simulink model "Demo_TrafficFlow_cs"  (Simulink 7.6)
settings: Solver=ode5  SolverMode=SingleTasking  StartTime=0.0  StopTime=60  FixedStep=1/20  RelTol=1e-3
PreLoadFcn:
  | check_NUTS_defined
  | try
  |     evalin('base', 'NUTS_defined;');
  | catch
  |     warningMsg = sprintf('PreScan Warning: \nUsing this model requires that MATLAB is configured for PreScan. The model may not display correctly.\n\n ==> Please open MATLAB with the dedicated buttons provided with PreScan');
  |     disp(warningMsg)
  |     warndlg(warningMsg,'WindowStyle','Modal');
  | end
  | 
  | model_closed = 0;
  | assignin('base',['model_closed_' bdroot],false);
InitFcn:
  | PreScanExperimentInitFcn(bdroot)
StopFcn:
  | PreScanExperimentStopFcn(bdroot)
undefined symbols (not defined in the file or in its folder): Advised_Speed_L1, Advised_Speed_L2, Advised_Speed_L3, DiscIntNeverNeededParam, spare_10, spare_5, spare_6, spare_7, spare_8, spare_9, State_L2, Throughput_L1, Throughput_L2, Throughput_L3, Velocity_L1, Velocity_L2, Velocity_L3
libraries: simulink x58, PreScanSimulinkLibrary x40, simulink_extras x32, PreScanUsefulBlocks x22, simulink_need_slupdate x3
(part 2 of 20)

== system Demo_TrafficFlow_cs/Antenna_Rx_2 ==
  Selector : Selector  IndexMode=Zero-based  IndexOptions=Starting index (dialog)  Indices=PortIndex  OutputSizes=PortWidth(1)
  U : Outport
  wires:
  Selector/1 -> U/1
  [SimCoreSensors] -> Selector/1

== system Demo_TrafficFlow_cs/Antenna_Rx_3 ==
  Selector : Selector  IndexMode=Zero-based  IndexOptions=Starting index (dialog)  Indices=PortIndex  OutputSizes=PortWidth(1)
  U : Outport
  wires:
  Selector/1 -> U/1
  [SimCoreSensors] -> Selector/1

== system Demo_TrafficFlow_cs/Antenna_Rx_4 ==
  Selector : Selector  IndexMode=Zero-based  IndexOptions=Starting index (dialog)  Indices=PortIndex  OutputSizes=PortWidth(1)
  U : Outport
  wires:
  Selector/1 -> U/1
  [SimCoreSensors] -> Selector/1

== system Demo_TrafficFlow_cs/Antenna_Rx_7 ==
  Selector : Selector  IndexMode=Zero-based  IndexOptions=Starting index (dialog)  Indices=PortIndex  OutputSizes=PortWidth(1)
  U : Outport
  wires:
  Selector/1 -> U/1
  [SimCoreSensors] -> Selector/1

== system Demo_TrafficFlow_cs/Antenna_Rx_8 ==
  Selector : Selector  IndexMode=Zero-based  IndexOptions=Starting index (dialog)  Indices=PortIndex  OutputSizes=PortWidth(1)
  U : Outport
  wires:
  Selector/1 -> U/1
  [SimCoreSensors] -> Selector/1

== system Demo_TrafficFlow_cs/Antenna_Rx_9 ==
  Selector : Selector  IndexMode=Zero-based  IndexOptions=Starting index (dialog)  Indices=PortIndex  OutputSizes=PortWidth(1)
  U : Outport
  wires:
  Selector/1 -> U/1
  [SimCoreSensors] -> Selector/1

== system Demo_TrafficFlow_cs/Antenna_Tx_2 ==
  Y : Inport
  Assignment : Assignment  IndexMode=Zero-based  SampleTime=TimeStep  IndexOptions=Starting index (dialog)  Indices=PortIndex  OutputSizes=[1 1]
  Data Store Read : DataStoreRead  DataStoreName=SimCoreActuators  SampleTime=TimeStep
  Data Store Write : DataStoreWrite  DataStoreName=SimCoreActuators
  wires:
  Assignment/1 -> Data Store Write/1
  Data Store Read/1 -> Assignment/1
  Y/1 -> Assignment/2

== system Demo_TrafficFlow_cs/Antenna_Tx_3 ==
  Y : Inport
  Assignment : Assignment  IndexMode=Zero-based  SampleTime=TimeStep  IndexOptions=Starting index (dialog)  Indices=PortIndex  OutputSizes=[1 1]
  Data Store Read : DataStoreRead  DataStoreName=SimCoreActuators  SampleTime=TimeStep
  Data Store Write : DataStoreWrite  DataStoreName=SimCoreActuators
  wires:
  Assignment/1 -> Data Store Write/1
  Data Store Read/1 -> Assignment/1
  Y/1 -> Assignment/2

== system Demo_TrafficFlow_cs/Antenna_Tx_4 ==
  Y : Inport
  Assignment : Assignment  IndexMode=Zero-based  SampleTime=TimeStep  IndexOptions=Starting index (dialog)  Indices=PortIndex  OutputSizes=[1 1]
  Data Store Read : DataStoreRead  DataStoreName=SimCoreActuators  SampleTime=TimeStep
  Data Store Write : DataStoreWrite  DataStoreName=SimCoreActuators
  wires:
  Assignment/1 -> Data Store Write/1
  Data Store Read/1 -> Assignment/1
  Y/1 -> Assignment/2

== system Demo_TrafficFlow_cs/Antenna_Tx_7 ==
  Y : Inport
  Assignment : Assignment  IndexMode=Zero-based  SampleTime=TimeStep  IndexOptions=Starting index (dialog)  Indices=PortIndex  OutputSizes=[1 1]
  Data Store Read : DataStoreRead  DataStoreName=SimCoreActuators  SampleTime=TimeStep
  Data Store Write : DataStoreWrite  DataStoreName=SimCoreActuators
  wires:
  Assignment/1 -> Data Store Write/1
  Data Store Read/1 -> Assignment/1
  Y/1 -> Assignment/2

== system Demo_TrafficFlow_cs/Antenna_Tx_8 ==
  Y : Inport
  Assignment : Assignment  IndexMode=Zero-based  SampleTime=TimeStep  IndexOptions=Starting index (dialog)  Indices=PortIndex  OutputSizes=[1 1]
  Data Store Read : DataStoreRead  DataStoreName=SimCoreActuators  SampleTime=TimeStep
  Data Store Write : DataStoreWrite  DataStoreName=SimCoreActuators
  wires:
  Assignment/1 -> Data Store Write/1
  Data Store Read/1 -> Assignment/1
  Y/1 -> Assignment/2

== system Demo_TrafficFlow_cs/Antenna_Tx_9 ==
  Y : Inport
  Assignment : Assignment  IndexMode=Zero-based  SampleTime=TimeStep  IndexOptions=Starting index (dialog)  Indices=PortIndex  OutputSizes=[1 1]
  Data Store Read : DataStoreRead  DataStoreName=SimCoreActuators  SampleTime=TimeStep
  Data Store Write : DataStoreWrite  DataStoreName=SimCoreActuators
  wires:
  Assignment/1 -> Data Store Write/1
  Data Store Read/1 -> Assignment/1
  Y/1 -> Assignment/2

== system Demo_TrafficFlow_cs/Audi_A8_1 ==
  note: Simple Longitudinal Advanced Cruise Control
      | with the following inputs:
      | - Advised  velocity received via Beacon
      | - Range to vehicle in front detected by AIR sensor
  note: Trajectories:
      | - Following path with ACC Velocity
      | - Looping trajectory (Reinsert vehicle on
      |   LANE 1 when triggered by Traffic Controller)
      | - Lane change is randomly executed
      |   when LANE 2 is open for traffic of LANE 1
      |   (misuse of Beacon, as visual interpretation
      |   of matrix sign is not possible)
  SELF_Audi_A8_1 : Inport  Tag=SELFInport
  RFOBU_1_Rx : Inport  Tag=OBURxInport  Port=2
  AIR_1 : Inport  Tag=AIRInport  Port=3  OutDataTypeStr=Bus: PRESCAN_AIRSENSORMESSAGE
  AIR_1_Demux : Subsystem  Tag=DemuxAIR  mask(PreScan Demux Block: AIR){DetectionType=BBox, detectableObjects=10}
      mask prompts: DetectionType "Detection Type"; detectableObjects "Max. detectable objects"
      mask help:
      | The Actor Information Receiver sensor provides a fast, simple and generic detection solution which is not tied up to a specific technology and can be used for scenarios in which a simple sensor is needed.
      |
      | For more information, please click Help.
      mask init:
      | switch DetectionType
      |     case 1
      |         DetectionTypeStr = 'Center';
      |     case 2
      |         DetectionTypeStr = 'BBox';
      |     case 3
      |         DetectionTypeStr = 'CoG';
      |     otherwise
      |         DetectionTypeStr = 'Unknown';
      | end
      settings: DeleteFcn=PreScanBlockDeleteFcn;
      mask Help: eval('openHelp(411)')
      mask StyleString: popup(Center|BBox|CoG),edit
      mask Display:
      | disp(char('Det. type:         ',['{\it',DetectionTypeStr,'}']),'texmode','on')
      | port_label('output',1,'Range [m]')
      | port_label('output',2,'{\theta} [deg]','texmode','on')
      | port_label('output',3,'{\phi} [deg]','texmode','on')
      | port_label('output',4,'ID [-]','texmode','on')
      | port_label('output',5,'Velocity [m/s]','texmode','on')
      | port_label('output',6,'Heading [deg]','texmode','on')
  Ground_{1..16} (16 alike) : Ground
  Memory : Memory
  Memory1 : Memory
  Memory2 : Memory
  RFOBU_1_Rx_Demux : Subsystem  Tag=DemuxOBURx  mask(PreScan Demux Block:){Technology='RF'}
      mask init:
      | IconTxt = char('','','','Technology:            ',['{\it',Technology,'}']);
      settings: DeleteFcn=PreScanBlockDeleteFcn;
      mask Display:
      | disp(IconTxt,'texmode','on')
      | port_label('output', 1, 'Beacon ID [-]')
      | port_label('output', 2, 'Beacon GPS Lat [deg]')
      | port_label('output', 3, 'Beacon GPS Lon [deg]')
      | port_label('output', 4, 'Beacon GPS Alt [m]')
      | port_label('output', 5, 'BeaconType [-]')
      | port_label('output', 6, 'Advised_Velocity')
      | port_label('output', 7, 'CarID_Lane1')
      | port_label('output', 8, 'CarID_Lane3')
      | port_label('output', 9, 'State_Lane2')
      | port_label('output', 10, 'spare_5')
      | port_label('output', 11, 'spare_6')
      | port_label('output', 12, 'spare_7')
      | port_label('output', 13, 'spare_8')
      | port_label('output', 14, 'spare_9')
      | port_label('output', 15, 'spare_10')
      | port_label('output', 16, 'spare_11')
      | port_label('output', 17, 'spare_12')
      | port_label('output', 18, 'spare_13')
      | port_label('output', 19, 'spare_14')
      | port_label('output', 20, 'spare_15')
  RFOBU_1_Tx_Mux : Subsystem  Tag=MuxOBUTx  mask(PreScan Mux Block:){Technology='RF'}
      mask init:
      | IconTxt = char('','','              Technology:',['{\it                ',Technology,'}']);
      settings: DeleteFcn=PreScanBlockDeleteFcn;
      mask Display:
      | disp(IconTxt,'texmode','on')
      | port_label('input', 1, 'Vehicle Type [-]')
      | port_label('input', 2, 'spare_1')
      | port_label('input', 3, 'spare_2')
      | port_label('input', 4, 'spare_3')
      | port_label('input', 5, 'spare_4')
      | port_label('input', 6, 'spare_5')
      | port_label('input', 7, 'spare_6')
      | port_label('input', 8, 'spare_7')
      | port_label('input', 9, 'spare_8')
      | port_label('input', 10, 'spare_9')
      | port_label('input', 11, 'spare_10')
      | port_label('input', 12, 'spare_11')
      | port_label('input', 13, 'spare_12')
      | port_label('input', 14, 'spare_13')
      | port_label('input', 15, 'spare_14')
      | port_label('input', 16, 'spare_15')
  SELF_Demux : Subsystem  Tag=DemuxSELF
      settings: DeleteFcn=PreScanBlockDeleteFcn;
      mask Type: PreScan Demux Block:
      mask Display:
      | port_label('output',1,'x [m]')
      | port_label('output',2,'y [m]')
      | port_label('output',3,'z [m]','texmode','on')
      | port_label('output',4,'Rotx [deg]','texmode','on')
      | port_label('output',5,'Roty [deg]','texmode','on')
      | port_label('output',6,'Rotz [deg]','texmode','on')
      | port_label('output',7,'GPS lat [deg]','texmode','on')
      | port_label('output',8,'GPS long [deg]','texmode','on')
      | port_label('output',9,'GPS alt [m]','texmode','on')
      | port_label('output',10,'Velocity [m/s]')
      | port_label('output',11,'Heading [deg]')
      | port_label('output',12,'Yaw rate [deg/s]')
  Selector1 : Selector  InputPortWidth=2  IndexOptions=Index vector (dialog)  Indices=[1]  OutputSizes=1
  Selector2 : Selector  InputPortWidth=2  IndexOptions=Index vector (dialog)  Indices=[1]  OutputSizes=1
  Selector3 : Selector  InputPortWidth=2  IndexOptions=Index vector (dialog)  Indices=[1]  OutputSizes=1
  Selector4 : Selector  InputPortWidth=10  IndexOptions=Index vector (dialog)  Indices=[1]  OutputSizes=1
  Selector5 : Selector  InputPortWidth=10  IndexOptions=Index vector (dialog)  Indices=[1]  OutputSizes=1
  Simple_Longitudinal_ACC : Subsystem
  Terminator1 : Terminator
  Terminator2 : Terminator
  Terminator_{1..31} (31 alike) : Terminator
  Trajectories : Subsystem  Tag=Trajectories
      mask Display: image(imread('Trajectory.png'));
  STATE_Audi_A8_1 : Outport  Tag=StateOutport
  RFOBU_1_Tx : Outport  Tag=AntennaTOutport  Port=2
  wires:
  Selector1/1 -> Memory/1
  Selector2/1 -> Memory2/1
  Selector3/1 -> Memory1/1
  Memory2/1 -> Simple_Longitudinal_ACC/1(Advised Velocity [km/h])
  Selector4/1 -> Simple_Longitudinal_ACC/2(Range [m])
  Selector5/1 -> Simple_Longitudinal_ACC/3(Velocity [m/s])
  Memory1/1 -> [state_lane2]
  Memory/1 -> [state_lane1]
  Simple_Longitudinal_ACC/1(ACC_Velocity [m/s]) -> [ACC_velocity]
  SELF_Audi_A8_1/1 -> SELF_Demux/1(SELF)
  RFOBU_1_Rx/1 -> RFOBU_1_Rx_Demux/1(Beacon_Rx)
  AIR_1/1 -> AIR_1_Demux/1(AIR)
  RFOBU_1_Tx_Mux/1(BeaconTx) -> RFOBU_1_Tx/1
  Trajectories/1(State) -> STATE_Audi_A8_1/1
  [ACC_velocity] -> Trajectories/1(Velocity)
  [state_lane1] -> Trajectories/2(OBUID_Reinsert [-])
  [state_lane2] -> Trajectories/3(StateLANE2 [-])
  RFOBU_1_Rx_Demux/8(spare_3) -> Terminator1/1
  RFOBU_1_Rx_Demux/9(spare_4) -> Selector3/1
  AIR_1_Demux/1(Range [m]) -> Selector4/1
  RFOBU_1_Rx_Demux/7(spare_2) -> Selector1/1
  RFOBU_1_Rx_Demux/6(spare_1) -> Selector2/1
  RFOBU_1_Rx_Demux/1(Beacon_ID) -> Terminator2/1
  AIR_1_Demux/5(Velocity [m/s]) -> Selector5/1
  AIR_1_Demux/2(Azimuth [deg]) -> Terminator_1/1
  AIR_1_Demux/3(Elevation [deg]) -> Terminator_2/1
  AIR_1_Demux/4(ID [-]) -> Terminator_3/1
  AIR_1_Demux/6(Heading [deg]) -> Terminator_4/1
  RFOBU_1_Rx_Demux/2(GPS_pos_lat_[deg]) -> Terminator_5/1
  RFOBU_1_Rx_Demux/3(GPS_pos_long_[deg]) -> Terminator_6/1
  RFOBU_1_Rx_Demux/4(GPS_pos_alt_[m]) -> Terminator_7/1
  RFOBU_1_Rx_Demux/5(BeaconType [-]) -> Terminator_8/1
  RFOBU_1_Rx_Demux/10(spare_5) -> Terminator_9/1
  RFOBU_1_Rx_Demux/11(spare_6) -> Terminator_10/1
  RFOBU_1_Rx_Demux/12(spare_7) -> Terminator_11/1
  RFOBU_1_Rx_Demux/13(spare_8) -> Terminator_12/1
  RFOBU_1_Rx_Demux/14(spare_9) -> Terminator_13/1
  RFOBU_1_Rx_Demux/15(spare_10) -> Terminator_14/1
  RFOBU_1_Rx_Demux/16(spare_11) -> Terminator_15/1
  RFOBU_1_Rx_Demux/17(spare_12) -> Terminator_16/1
  RFOBU_1_Rx_Demux/18(spare_13) -> Terminator_17/1
  RFOBU_1_Rx_Demux/19(spare_14) -> Terminator_18/1
  RFOBU_1_Rx_Demux/20(spare_15) -> Terminator_19/1
  Ground_1/1 -> RFOBU_1_Tx_Mux/1(Vehicle_Type)
  Ground_2/1 -> RFOBU_1_Tx_Mux/2(spare_1)
  Ground_3/1 -> RFOBU_1_Tx_Mux/3(spare_2)
  Ground_4/1 -> RFOBU_1_Tx_Mux/4(spare_3)
  Ground_5/1 -> RFOBU_1_Tx_Mux/5(spare_4)
  Ground_6/1 -> RFOBU_1_Tx_Mux/6(spare_5)
  Ground_7/1 -> RFOBU_1_Tx_Mux/7(spare_6)
  Ground_8/1 -> RFOBU_1_Tx_Mux/8(spare_7)
  Ground_9/1 -> RFOBU_1_Tx_Mux/9(spare_8)
  Ground_10/1 -> RFOBU_1_Tx_Mux/10(spare_9)
  Ground_11/1 -> RFOBU_1_Tx_Mux/11(spare_10)
  Ground_12/1 -> RFOBU_1_Tx_Mux/12(spare_11)
  Ground_13/1 -> RFOBU_1_Tx_Mux/13(spare_12)
  Ground_14/1 -> RFOBU_1_Tx_Mux/14(spare_13)
  Ground_15/1 -> RFOBU_1_Tx_Mux/15(spare_14)
  Ground_16/1 -> RFOBU_1_Tx_Mux/16(spare_15)
  SELF_Demux/1(x [m]) -> Terminator_20/1
  SELF_Demux/2(y [m]) -> Terminator_21/1
  SELF_Demux/3(z [m]) -> Terminator_22/1
  SELF_Demux/4(rotx [deg]) -> Terminator_23/1
  SELF_Demux/5(roty [deg]) -> Terminator_24/1
  SELF_Demux/6(rotz [deg]) -> Terminator_25/1
  SELF_Demux/7(GPS lat [deg]) -> Terminator_26/1
  SELF_Demux/8(GPS long [deg]) -> Terminator_27/1
  SELF_Demux/9(GPS alt [m]) -> Terminator_28/1
  SELF_Demux/10(velocity [m/s]) -> Terminator_29/1
  SELF_Demux/11(heading [deg]) -> Terminator_30/1
  SELF_Demux/12(yaw rate [deg/s]) -> Terminator_31/1

== system Demo_TrafficFlow_cs/BMW_X5_1 ==
  note: Simple Longitudinal Advanced Cruise Control
      | with the following inputs:
      | - Advised  velocity received via Beacon
      | - Range to vehicle in front detected by AIR sensor
  note: Trajectories:
      | - Following path with ACC Velocity
      | - Looping trajectory (Reinsert vehicle on
      |   LANE 1 when triggered by Traffic Controller)
      | - Lane change is randomly executed
      |   when LANE 2 is open for traffic of LANE 1
      |   (misuse of Beacon, as visual interpretation
      |   of matrix sign is not possible)
  SELF_BMW_X5_1 : Inport  Tag=SELFInport
  RFOBU_2_Rx : Inport  Tag=OBURxInport  Port=2
  AIR_2 : Inport  Tag=AIRInport  Port=3  OutDataTypeStr=Bus: PRESCAN_AIRSENSORMESSAGE
  AIR_2_Demux : Subsystem  Tag=DemuxAIR  mask(PreScan Demux Block: AIR){DetectionType=BBox, detectableObjects=10}
      mask prompts: DetectionType "Detection Type"; detectableObjects "Max. detectable objects"
      mask help:
      | The Actor Information Receiver sensor provides a fast, simple and generic detection solution which is not tied up to a specific technology and can be used for scenarios in which a simple sensor is needed.
      |
      | For more information, please click Help.
      mask init: same as Demo_TrafficFlow_cs/Audi_A8_1/AIR_1_Demux
      settings: DeleteFcn=PreScanBlockDeleteFcn;
      mask Help: eval('openHelp(411)')
      mask StyleString: popup(Center|BBox|CoG),edit
      mask Display:
      | disp(char('Det. type:         ',['{\it',DetectionTypeStr,'}']),'texmode','on')
      | port_label('output',1,'Range [m]')
      | port_label('output',2,'{\theta} [deg]','texmode','on')
      | port_label('output',3,'{\phi} [deg]','texmode','on')
      | port_label('output',4,'ID [-]','texmode','on')
      | port_label('output',5,'Velocity [m/s]','texmode','on')
      | port_label('output',6,'Heading [deg]','texmode','on')
  Ground_{1..16} (16 alike) : Ground
  Memory : Memory
  Memory1 : Memory
  Memory2 : Memory
  RFOBU_2_Rx_Demux : Subsystem  Tag=DemuxOBURx  mask(PreScan Demux Block:){Technology='RF'}
      mask init:
      | IconTxt = char('','','','Technology:            ',['{\it',Technology,'}']);
      settings: DeleteFcn=PreScanBlockDeleteFcn;
      mask Display:
      | disp(IconTxt,'texmode','on')
      | port_label('output', 1, 'Beacon ID [-]')
      | port_label('output', 2, 'Beacon GPS Lat [deg]')
      | port_label('output', 3, 'Beacon GPS Lon [deg]')
      | port_label('output', 4, 'Beacon GPS Alt [m]')
      | port_label('output', 5, 'BeaconType [-]')
      | port_label('output', 6, 'Advised_Velocity')
      | port_label('output', 7, 'CarID_Lane1')
      | port_label('output', 8, 'CarID_Lane3')
      | port_label('output', 9, 'State_Lane2')
      | port_label('output', 10, 'spare_5')
      | port_label('output', 11, 'spare_6')
      | port_label('output', 12, 'spare_7')
      | port_label('output', 13, 'spare_8')
      | port_label('output', 14, 'spare_9')
      | port_label('output', 15, 'spare_10')
      | port_label('output', 16, 'spare_11')
      | port_label('output', 17, 'spare_12')
      | port_label('output', 18, 'spare_13')
      | port_label('output', 19, 'spare_14')
      | port_label('output', 20, 'spare_15')
  RFOBU_2_Tx_Mux : Subsystem  Tag=MuxOBUTx  mask(PreScan Mux Block:){Technology='RF'}
      mask init:
      | IconTxt = char('','','              Technology:',['{\it                ',Technology,'}']);
      settings: DeleteFcn=PreScanBlockDeleteFcn;
      mask Display:
      | disp(IconTxt,'texmode','on')
      | port_label('input', 1, 'Vehicle Type [-]')
      | port_label('input', 2, 'spare_1')
      | port_label('input', 3, 'spare_2')
      | port_label('input', 4, 'spare_3')
      | port_label('input', 5, 'spare_4')
      | port_label('input', 6, 'spare_5')
      | port_label('input', 7, 'spare_6')
      | port_label('input', 8, 'spare_7')
      | port_label('input', 9, 'spare_8')
      | port_label('input', 10, 'spare_9')
      | port_label('input', 11, 'spare_10')
      | port_label('input', 12, 'spare_11')
      | port_label('input', 13, 'spare_12')
      | port_label('input', 14, 'spare_13')
      | port_label('input', 15, 'spare_14')
      | port_label('input', 16, 'spare_15')
  SELF_Demux : Subsystem  Tag=DemuxSELF
      settings: DeleteFcn=PreScanBlockDeleteFcn;
      mask Type: PreScan Demux Block:
      mask Display:
      | port_label('output',1,'x [m]')
      | port_label('output',2,'y [m]')
      | port_label('output',3,'z [m]','texmode','on')
      | port_label('output',4,'Rotx [deg]','texmode','on')
      | port_label('output',5,'Roty [deg]','texmode','on')
      | port_label('output',6,'Rotz [deg]','texmode','on')
      | port_label('output',7,'GPS lat [deg]','texmode','on')
      | port_label('output',8,'GPS long [deg]','texmode','on')
      | port_label('output',9,'GPS alt [m]','texmode','on')
      | port_label('output',10,'Velocity [m/s]')
      | port_label('output',11,'Heading [deg]')
      | port_label('output',12,'Yaw rate [deg/s]')
  Selector1 : Selector  InputPortWidth=2  IndexOptions=Index vector (dialog)  Indices=[1]  OutputSizes=1
  Selector2 : Selector  InputPortWidth=2  IndexOptions=Index vector (dialog)  Indices=[1]  OutputSizes=1
  Selector3 : Selector  InputPortWidth=2  IndexOptions=Index vector (dialog)  Indices=[1]  OutputSizes=1
  Selector4 : Selector  InputPortWidth=10  IndexOptions=Index vector (dialog)  Indices=[1]  OutputSizes=1
  Selector5 : Selector  InputPortWidth=10  IndexOptions=Index vector (dialog)  Indices=[1]  OutputSizes=1
  Simple_Longitudinal_ACC : Subsystem
  Terminator1 : Terminator
  Terminator2 : Terminator
  Terminator_{1..31} (31 alike) : Terminator
  Trajectories : Subsystem  Tag=Trajectories
      mask Display: image(imread('Trajectory.png'));
  STATE_BMW_X5_1 : Outport  Tag=StateOutport
  RFOBU_2_Tx : Outport  Tag=AntennaTOutport  Port=2
  wires:
  Selector1/1 -> Memory/1
  Selector2/1 -> Memory2/1
  Selector3/1 -> Memory1/1
  Selector4/1 -> Simple_Longitudinal_ACC/2(Range [m])
  Memory2/1 -> Simple_Longitudinal_ACC/1(Advised Velocity [km/h])
  Selector5/1 -> Simple_Longitudinal_ACC/3(Velocity [m/s])
  Memory1/1 -> [state_lane2]
  Memory/1 -> [state_lane3]
  Simple_Longitudinal_ACC/1(ACC_Velocity [m/s]) -> [ACC_velocity]
  SELF_BMW_X5_1/1 -> SELF_Demux/1(SELF)
  RFOBU_2_Rx/1 -> RFOBU_2_Rx_Demux/1(Beacon_Rx)
  AIR_2/1 -> AIR_2_Demux/1(AIR)
  RFOBU_2_Tx_Mux/1(BeaconTx) -> RFOBU_2_Tx/1
  Trajectories/1(State) -> STATE_BMW_X5_1/1
  [ACC_velocity] -> Trajectories/1(Velocity)
  [state_lane3] -> Trajectories/2(OBUID_Reinsert [-])
  [state_lane2] -> Trajectories/3(StateLANE2 [-])
  RFOBU_2_Rx_Demux/7(spare_2) -> Terminator1/1
  RFOBU_2_Rx_Demux/9(spare_4) -> Selector3/1
  RFOBU_2_Rx_Demux/8(spare_3) -> Selector1/1
  RFOBU_2_Rx_Demux/6(spare_1) -> Selector2/1
  AIR_2_Demux/1(Range [m]) -> Selector4/1
  RFOBU_2_Rx_Demux/1(Beacon_ID) -> Terminator2/1
  AIR_2_Demux/5(Velocity [m/s]) -> Selector5/1
  AIR_2_Demux/2(Azimuth [deg]) -> Terminator_1/1
  AIR_2_Demux/3(Elevation [deg]) -> Terminator_2/1
  AIR_2_Demux/4(ID [-]) -> Terminator_3/1
  AIR_2_Demux/6(Heading [deg]) -> Terminator_4/1
  RFOBU_2_Rx_Demux/2(GPS_pos_lat_[deg]) -> Terminator_5/1
  RFOBU_2_Rx_Demux/3(GPS_pos_long_[deg]) -> Terminator_6/1
  RFOBU_2_Rx_Demux/4(GPS_pos_alt_[m]) -> Terminator_7/1
  RFOBU_2_Rx_Demux/5(BeaconType [-]) -> Terminator_8/1
  RFOBU_2_Rx_Demux/10(spare_5) -> Terminator_9/1
  RFOBU_2_Rx_Demux/11(spare_6) -> Terminator_10/1
  RFOBU_2_Rx_Demux/12(spare_7) -> Terminator_11/1
  RFOBU_2_Rx_Demux/13(spare_8) -> Terminator_12/1
  RFOBU_2_Rx_Demux/14(spare_9) -> Terminator_13/1
  RFOBU_2_Rx_Demux/15(spare_10) -> Terminator_14/1
  RFOBU_2_Rx_Demux/16(spare_11) -> Terminator_15/1
  RFOBU_2_Rx_Demux/17(spare_12) -> Terminator_16/1
  RFOBU_2_Rx_Demux/18(spare_13) -> Terminator_17/1
  RFOBU_2_Rx_Demux/19(spare_14) -> Terminator_18/1
  RFOBU_2_Rx_Demux/20(spare_15) -> Terminator_19/1
  Ground_1/1 -> RFOBU_2_Tx_Mux/1(Vehicle_Type)
  Ground_2/1 -> RFOBU_2_Tx_Mux/2(spare_1)
  Ground_3/1 -> RFOBU_2_Tx_Mux/3(spare_2)
  Ground_4/1 -> RFOBU_2_Tx_Mux/4(spare_3)
  Ground_5/1 -> RFOBU_2_Tx_Mux/5(spare_4)
  Ground_6/1 -> RFOBU_2_Tx_Mux/6(spare_5)
  Ground_7/1 -> RFOBU_2_Tx_Mux/7(spare_6)
  Ground_8/1 -> RFOBU_2_Tx_Mux/8(spare_7)
  Ground_9/1 -> RFOBU_2_Tx_Mux/9(spare_8)
  Ground_10/1 -> RFOBU_2_Tx_Mux/10(spare_9)
  Ground_11/1 -> RFOBU_2_Tx_Mux/11(spare_10)
  Ground_12/1 -> RFOBU_2_Tx_Mux/12(spare_11)
  Ground_13/1 -> RFOBU_2_Tx_Mux/13(spare_12)
  Ground_14/1 -> RFOBU_2_Tx_Mux/14(spare_13)
  Ground_15/1 -> RFOBU_2_Tx_Mux/15(spare_14)
  Ground_16/1 -> RFOBU_2_Tx_Mux/16(spare_15)
  SELF_Demux/1(x [m]) -> Terminator_20/1
  SELF_Demux/2(y [m]) -> Terminator_21/1
  SELF_Demux/3(z [m]) -> Terminator_22/1
  SELF_Demux/4(rotx [deg]) -> Terminator_23/1
  SELF_Demux/5(roty [deg]) -> Terminator_24/1
  SELF_Demux/6(rotz [deg]) -> Terminator_25/1
  SELF_Demux/7(GPS lat [deg]) -> Terminator_26/1
  SELF_Demux/8(GPS long [deg]) -> Terminator_27/1
  SELF_Demux/9(GPS alt [m]) -> Terminator_28/1
  SELF_Demux/10(velocity [m/s]) -> Terminator_29/1
  SELF_Demux/11(heading [deg]) -> Terminator_30/1
  SELF_Demux/12(yaw rate [deg/s]) -> Terminator_31/1

== system Demo_TrafficFlow_cs/Citroen_C3_1 ==
  note: Simple Longitudinal Advanced Cruise Control
      | with the following inputs:
      | - Advised  velocity received via Beacon
      | - Range to vehicle in front detected by AIR sensor
  note: Trajectories:
      | - Following path with ACC Velocity
      | - Looping trajectory (Reinsert vehicle on
      |   LANE 1 when triggered by Traffic Controller)
      | - Lane change is randomly executed
      |   when LANE 2 is open for traffic of LANE 1
      |   (misuse of Beacon, as visual interpretation
      |   of matrix sign is not possible)
  SELF_Citroen_C3_1 : Inport  Tag=SELFInport
  RFOBU_3_Rx : Inport  Tag=OBURxInport  Port=2
  AIR_3 : Inport  Tag=AIRInport  Port=3  OutDataTypeStr=Bus: PRESCAN_AIRSENSORMESSAGE
  AIR_3_Demux : Subsystem  Tag=DemuxAIR  mask(PreScan Demux Block: AIR){DetectionType=BBox, detectableObjects=10}
      mask prompts: DetectionType "Detection Type"; detectableObjects "Max. detectable objects"
      mask help:
      | The Actor Information Receiver sensor provides a fast, simple and generic detection solution which is not tied up to a specific technology and can be used for scenarios in which a simple sensor is needed.
      |
      | For more information, please click Help.
      mask init: same as Demo_TrafficFlow_cs/Audi_A8_1/AIR_1_Demux
      settings: DeleteFcn=PreScanBlockDeleteFcn;
      mask Help: eval('openHelp(411)')
      mask StyleString: popup(Center|BBox|CoG),edit
      mask Display:
      | disp(char('Det. type:         ',['{\it',DetectionTypeStr,'}']),'texmode','on')
      | port_label('output',1,'Range [m]')
      | port_label('output',2,'{\theta} [deg]','texmode','on')
      | port_label('output',3,'{\phi} [deg]','texmode','on')
      | port_label('output',4,'ID [-]','texmode','on')
      | port_label('output',5,'Velocity [m/s]','texmode','on')
      | port_label('output',6,'Heading [deg]','texmode','on')
  Ground_{1..16} (16 alike) : Ground
  Memory : Memory
  Memory1 : Memory
  Memory2 : Memory
  RFOBU_3_Rx_Demux : Subsystem  Tag=DemuxOBURx  mask(PreScan Demux Block:){Technology='RF'}
      mask init:
      | IconTxt = char('','','','Technology:            ',['{\it',Technology,'}']);
      settings: DeleteFcn=PreScanBlockDeleteFcn;
      mask Display:
      | disp(IconTxt,'texmode','on')
      | port_label('output', 1, 'Beacon ID [-]')
      | port_label('output', 2, 'Beacon GPS Lat [deg]')
      | port_label('output', 3, 'Beacon GPS Lon [deg]')
      | port_label('output', 4, 'Beacon GPS Alt [m]')
      | port_label('output', 5, 'BeaconType [-]')
      | port_label('output', 6, 'Advised_Velocity')
      | port_label('output', 7, 'CarID_Lane1')
      | port_label('output', 8, 'CarID_Lane3')
      | port_label('output', 9, 'State_Lane2')
      | port_label('output', 10, 'spare_5')
      | port_label('output', 11, 'spare_6')
      | port_label('output', 12, 'spare_7')
      | port_label('output', 13, 'spare_8')
      | port_label('output', 14, 'spare_9')
      | port_label('output', 15, 'spare_10')
      | port_label('output', 16, 'spare_11')
      | port_label('output', 17, 'spare_12')
      | port_label('output', 18, 'spare_13')
      | port_label('output', 19, 'spare_14')
      | port_label('output', 20, 'spare_15')
  RFOBU_3_Tx_Mux : Subsystem  Tag=MuxOBUTx  mask(PreScan Mux Block:){Technology='RF'}
      mask init:
      | IconTxt = char('','','              Technology:',['{\it                ',Technology,'}']);
      settings: DeleteFcn=PreScanBlockDeleteFcn;
      mask Display:
      | disp(IconTxt,'texmode','on')
      | port_label('input', 1, 'Vehicle Type [-]')
      | port_label('input', 2, 'spare_1')
      | port_label('input', 3, 'spare_2')
      | port_label('input', 4, 'spare_3')
      | port_label('input', 5, 'spare_4')
      | port_label('input', 6, 'spare_5')
      | port_label('input', 7, 'spare_6')
      | port_label('input', 8, 'spare_7')
      | port_label('input', 9, 'spare_8')
      | port_label('input', 10, 'spare_9')
      | port_label('input', 11, 'spare_10')
      | port_label('input', 12, 'spare_11')
      | port_label('input', 13, 'spare_12')
      | port_label('input', 14, 'spare_13')
      | port_label('input', 15, 'spare_14')
      | port_label('input', 16, 'spare_15')
  SELF_Demux : Subsystem  Tag=DemuxSELF
      settings: DeleteFcn=PreScanBlockDeleteFcn;
      mask Type: PreScan Demux Block:
      mask Display:
      | port_label('output',1,'x [m]')
      | port_label('output',2,'y [m]')
      | port_label('output',3,'z [m]','texmode','on')
      | port_label('output',4,'Rotx [deg]','texmode','on')
      | port_label('output',5,'Roty [deg]','texmode','on')
      | port_label('output',6,'Rotz [deg]','texmode','on')
      | port_label('output',7,'GPS lat [deg]','texmode','on')
      | port_label('output',8,'GPS long [deg]','texmode','on')
      | port_label('output',9,'GPS alt [m]','texmode','on')
      | port_label('output',10,'Velocity [m/s]')
      | port_label('output',11,'Heading [deg]')
      | port_label('output',12,'Yaw rate [deg/s]')
  Selector1 : Selector  InputPortWidth=2  IndexOptions=Index vector (dialog)  Indices=[1]  OutputSizes=1
  Selector2 : Selector  InputPortWidth=2  IndexOptions=Index vector (dialog)  Indices=[1]  OutputSizes=1
  Selector3 : Selector  InputPortWidth=2  IndexOptions=Index vector (dialog)  Indices=[1]  OutputSizes=1
  Selector4 : Selector  InputPortWidth=10  IndexOptions=Index vector (dialog)  Indices=[1]  OutputSizes=1
  Selector5 : Selector  InputPortWidth=10  IndexOptions=Index vector (dialog)  Indices=[1]  OutputSizes=1
  Simple_Longitudinal_ACC : Subsystem
  Terminator_{1..33} (33 alike) : Terminator
  Trajectories : Subsystem  Tag=Trajectories
      settings: DeleteFcn=PreScanBlockDeleteFcn;
      mask Display: image(imread('Trajectory.png'));
  STATE_Citroen_C3_1 : Outport  Tag=StateOutport
  RFOBU_3_Tx : Outport  Tag=AntennaTOutport  Port=2
  wires:
  Selector1/1 -> Memory/1
  Selector2/1 -> Memory2/1
  Selector3/1 -> Memory1/1
  Memory2/1 -> Simple_Longitudinal_ACC/1(Advised Velocity [km/h])
  Selector4/1 -> Simple_Longitudinal_ACC/2(Range [m])
  Selector5/1 -> Simple_Longitudinal_ACC/3(Velocity [m/s])
  Memory/1 -> [state_lane1]
  Simple_Longitudinal_ACC/1(ACC_Velocity [m/s]) -> [ACC_velocity]
  Memory1/1 -> [state_lane2]
  SELF_Citroen_C3_1/1 -> SELF_Demux/1(SELF)
  RFOBU_3_Rx/1 -> RFOBU_3_Rx_Demux/1(Beacon_Rx)
  AIR_3/1 -> AIR_3_Demux/1(AIR)
  RFOBU_3_Tx_Mux/1(BeaconTx) -> RFOBU_3_Tx/1
  Trajectories/1(State) -> STATE_Citroen_C3_1/1
  [state_lane2] -> Trajectories/3(StateLANE2 [-])
  [state_lane1] -> Trajectories/2(OBUID_Reinsert [-])
  [ACC_velocity] -> Trajectories/1(Velocity)
  RFOBU_3_Rx_Demux/6(spare_1) -> Selector2/1
  RFOBU_3_Rx_Demux/7(spare_2) -> Selector1/1
  RFOBU_3_Rx_Demux/9(spare_4) -> Selector3/1
  AIR_3_Demux/1(Range [m]) -> Selector4/1
  AIR_3_Demux/5(Velocity [m/s]) -> Selector5/1
  AIR_3_Demux/2(Azimuth [deg]) -> Terminator_1/1
  AIR_3_Demux/3(Elevation [deg]) -> Terminator_2/1
  AIR_3_Demux/4(ID [-]) -> Terminator_3/1
  AIR_3_Demux/6(Heading [deg]) -> Terminator_4/1
  RFOBU_3_Rx_Demux/1(Beacon_ID) -> Terminator_5/1
  RFOBU_3_Rx_Demux/2(GPS_pos_lat_[deg]) -> Terminator_6/1
  RFOBU_3_Rx_Demux/3(GPS_pos_long_[deg]) -> Terminator_7/1
  RFOBU_3_Rx_Demux/4(GPS_pos_alt_[m]) -> Terminator_8/1
  RFOBU_3_Rx_Demux/5(BeaconType [-]) -> Terminator_9/1
  RFOBU_3_Rx_Demux/8(spare_3) -> Terminator_10/1
  RFOBU_3_Rx_Demux/10(spare_5) -> Terminator_11/1
  RFOBU_3_Rx_Demux/11(spare_6) -> Terminator_12/1
  RFOBU_3_Rx_Demux/12(spare_7) -> Terminator_13/1
  RFOBU_3_Rx_Demux/13(spare_8) -> Terminator_14/1
  RFOBU_3_Rx_Demux/14(spare_9) -> Terminator_15/1
  RFOBU_3_Rx_Demux/15(spare_10) -> Terminator_16/1
  RFOBU_3_Rx_Demux/16(spare_11) -> Terminator_17/1
  RFOBU_3_Rx_Demux/17(spare_12) -> Terminator_18/1
  RFOBU_3_Rx_Demux/18(spare_13) -> Terminator_19/1
  RFOBU_3_Rx_Demux/19(spare_14) -> Terminator_20/1
  RFOBU_3_Rx_Demux/20(spare_15) -> Terminator_21/1
  Ground_1/1 -> RFOBU_3_Tx_Mux/1(Vehicle_Type)
  Ground_2/1 -> RFOBU_3_Tx_Mux/2(spare_1)
  Ground_3/1 -> RFOBU_3_Tx_Mux/3(spare_2)
  Ground_4/1 -> RFOBU_3_Tx_Mux/4(spare_3)
  Ground_5/1 -> RFOBU_3_Tx_Mux/5(spare_4)
  Ground_6/1 -> RFOBU_3_Tx_Mux/6(spare_5)
  Ground_7/1 -> RFOBU_3_Tx_Mux/7(spare_6)
  Ground_8/1 -> RFOBU_3_Tx_Mux/8(spare_7)
  Ground_9/1 -> RFOBU_3_Tx_Mux/9(spare_8)
  Ground_10/1 -> RFOBU_3_Tx_Mux/10(spare_9)
  Ground_11/1 -> RFOBU_3_Tx_Mux/11(spare_10)
  Ground_12/1 -> RFOBU_3_Tx_Mux/12(spare_11)
  Ground_13/1 -> RFOBU_3_Tx_Mux/13(spare_12)
  Ground_14/1 -> RFOBU_3_Tx_Mux/14(spare_13)
  Ground_15/1 -> RFOBU_3_Tx_Mux/15(spare_14)
  Ground_16/1 -> RFOBU_3_Tx_Mux/16(spare_15)
  SELF_Demux/1(x [m]) -> Terminator_22/1
  SELF_Demux/2(y [m]) -> Terminator_23/1
  SELF_Demux/3(z [m]) -> Terminator_24/1
  SELF_Demux/4(rotx [deg]) -> Terminator_25/1
  SELF_Demux/5(roty [deg]) -> Terminator_26/1
  SELF_Demux/6(rotz [deg]) -> Terminator_27/1
  SELF_Demux/7(GPS lat [deg]) -> Terminator_28/1
  SELF_Demux/8(GPS long [deg]) -> Terminator_29/1
  SELF_Demux/9(GPS alt [m]) -> Terminator_30/1
  SELF_Demux/10(velocity [m/s]) -> Terminator_31/1
  SELF_Demux/11(heading [deg]) -> Terminator_32/1
  SELF_Demux/12(yaw rate [deg/s]) -> Terminator_33/1

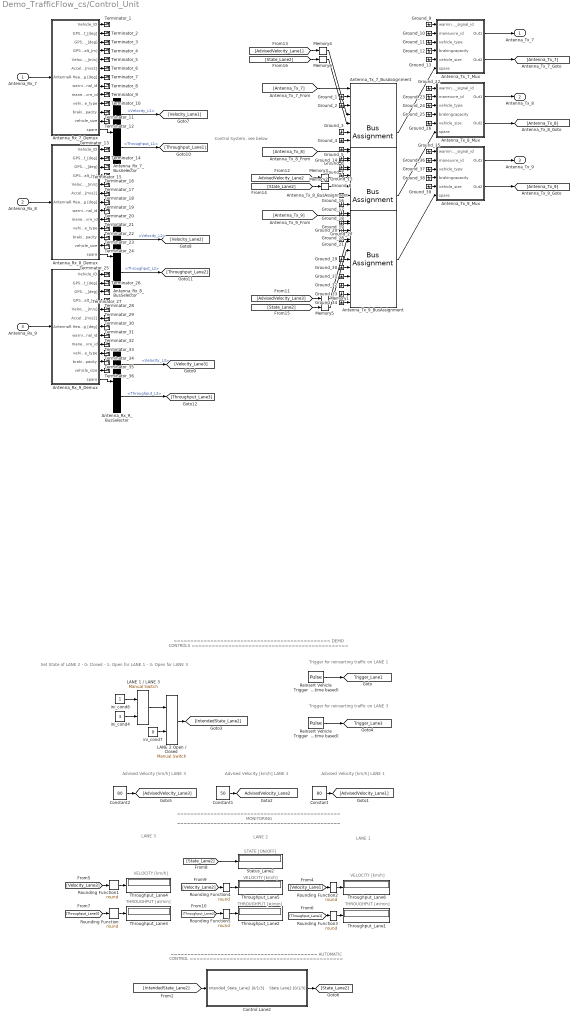
== system Demo_TrafficFlow_cs/Control_Unit ==
  note: Control System, see below
  note: LANE 1
  note: LANE 3
  note: LANE 2
  note: VELOCITY [km/h]
  note: THROUGHPUT [#/min]
  note: ================================================= MONITORING =================================================
  note: ============================================ AUTOMATIC CONTROL ==============================================
  note: =============================================== DEMO CONTROLS ===============================================
  note: STATE [ON/OFF]
  note: VELOCITY [km/h]
  note: THROUGHPUT [#/min]
  note: VELOCITY [km/h]
  note: THROUGHPUT [#/min]
  note: Trigger for reinserting traffic on LANE 1
  note: Trigger for reinserting traffic on LANE 3
  note: Set State of LANE 2
      | - 0: Closed
      | - 1: Open for LANE 1
      | - 3: Open for LANE 3
  note: Advised Velocity [km/h] LANE 1
  note: Advised Velocity [km/h] LANE 2
  note: Advised Velocity [km/h] LANE 3
  Antenna_Rx_7 : Inport  Tag=AntennaRInport
  Antenna_Rx_8 : Inport  Tag=AntennaRInport  Port=2
  Antenna_Rx_9 : Inport  Tag=AntennaRInport  Port=3
  Antenna_Rx_7_BusSelector : BusSelector  Tag=BusAntennaR  OutputSignals=Velocity_L1,Throughput_L1
  Antenna_Rx_7_Demux : Subsystem  Tag=DemuxAntennaR  mask(PreScan Demux Block:){Channel=1}
      settings: DeleteFcn=PreScanBlockDeleteFcn;
      mask Display:
      | fprintf('Channel: %d',Channel)
      | port_label('output', 1, 'Vehicle_ID')
      | port_label('output', 2, 'GPS Pos Lat [deg]')
      | port_label('output', 3, 'GPS Pos Long [deg]')
      | port_label('output', 4, 'GPS Pos Alt [m]')
      | port_label('output', 5, 'Velocity [m/s]')
      | port_label('output', 6, 'Acceleration [m/s2]')
      | port_label('output', 7, 'Heading [deg]')
      | port_label('output', 8, 'Warning signal ID')
      | port_label('output', 9, 'Maneuvre ID')
      | port_label('output', 10, 'Vehicle Type')
      | port_label('output', 11, 'Braking Capacity')
      | port_label('output', 12, 'Vehicle Size')
      | port_label('output', 13, 'Spare')
  Antenna_Rx_8_BusSelector : BusSelector  Tag=BusAntennaR  OutputSignals=Velocity_L2,Throughput_L2
  Antenna_Rx_8_Demux : Subsystem  Tag=DemuxAntennaR  mask(PreScan Demux Block:){Channel=2}
      settings: DeleteFcn=PreScanBlockDeleteFcn;
      mask Display:
      | fprintf('Channel: %d',Channel)
      | port_label('output', 1, 'Vehicle_ID')
      | port_label('output', 2, 'GPS Pos Lat [deg]')
      | port_label('output', 3, 'GPS Pos Long [deg]')
      | port_label('output', 4, 'GPS Pos Alt [m]')
      | port_label('output', 5, 'Velocity [m/s]')
      | port_label('output', 6, 'Acceleration [m/s2]')
      | port_label('output', 7, 'Heading [deg]')
      | port_label('output', 8, 'Warning signal ID')
      | port_label('output', 9, 'Maneuvre ID')
      | port_label('output', 10, 'Vehicle Type')
      | port_label('output', 11, 'Braking Capacity')
      | port_label('output', 12, 'Vehicle Size')
      | port_label('output', 13, 'Spare')
  Antenna_Rx_9_BusSelector : BusSelector  Tag=BusAntennaR  OutputSignals=Velocity_L3,Throughput_L3
  Antenna_Rx_9_Demux : Subsystem  Tag=DemuxAntennaR  mask(PreScan Demux Block:){Channel=3}
      settings: DeleteFcn=PreScanBlockDeleteFcn;
      mask Display:
      | fprintf('Channel: %d',Channel)
      | port_label('output', 1, 'Vehicle_ID')
      | port_label('output', 2, 'GPS Pos Lat [deg]')
      | port_label('output', 3, 'GPS Pos Long [deg]')
      | port_label('output', 4, 'GPS Pos Alt [m]')
      | port_label('output', 5, 'Velocity [m/s]')
      | port_label('output', 6, 'Acceleration [m/s2]')
      | port_label('output', 7, 'Heading [deg]')
      | port_label('output', 8, 'Warning signal ID')
      | port_label('output', 9, 'Maneuvre ID')
      | port_label('output', 10, 'Vehicle Type')
      | port_label('output', 11, 'Braking Capacity')
      | port_label('output', 12, 'Vehicle Size')
      | port_label('output', 13, 'Spare')
  Antenna_Tx_7_BusAssignment : BusAssignment  Tag=BusAntennaT  AssignedSignals=Velocity_L1,Throughput_L1,Advised_Speed_L1,State_L2,spare_5,spare_6,spare_7,spare_8,spare_9,spare_10
  Antenna_Tx_7_Mux : Subsystem  Tag=MuxAntennaT  mask(PreScan Mux Block:){Channel=1, TimeStep=1/20}
      mask prompts: TimeStep "Time Step [s]"
      settings: DeleteFcn=PreScanBlockDeleteFcn;
      mask Display:
      | fprintf('Channel: %d',Channel)
      | port_label('input', 1, 'Warning signal ID')
      | port_label('input', 2, 'Maneuvre ID')
      | port_label('input', 3, 'Vehicle Type')
      | port_label('input', 4, 'Braking Capacity')
      | port_label('input', 5, 'Vehicle Size')
      | port_label('input', 6, 'spare')
  Antenna_Tx_8_BusAssignment : BusAssignment  Tag=BusAntennaT  AssignedSignals=Velocity_L2,Throughput_L2,Advised_Speed_L2,State_L2,spare_5,spare_6,spare_7,spare_8,spare_9,spare_10
  Antenna_Tx_8_Mux : Subsystem  Tag=MuxAntennaT  mask(PreScan Mux Block:){Channel=2, TimeStep=1/20}
      mask prompts: TimeStep "Time Step [s]"
      settings: DeleteFcn=PreScanBlockDeleteFcn;
      mask Display:
      | fprintf('Channel: %d',Channel)
      | port_label('input', 1, 'Warning signal ID')
      | port_label('input', 2, 'Maneuvre ID')
      | port_label('input', 3, 'Vehicle Type')
      | port_label('input', 4, 'Braking Capacity')
      | port_label('input', 5, 'Vehicle Size')
      | port_label('input', 6, 'spare')
  Antenna_Tx_9_BusAssignment : BusAssignment  Tag=BusAntennaT  AssignedSignals=Velocity_L3,Throughput_L3,Advised_Speed_L3,State_L2,spare_5,spare_6,spare_7,spare_8,spare_9,spare_10
  Antenna_Tx_9_Mux : Subsystem  Tag=MuxAntennaT  mask(PreScan Mux Block:){Channel=3, TimeStep=1/20}
      mask prompts: TimeStep "Time Step [s]"
      settings: DeleteFcn=PreScanBlockDeleteFcn;
      mask Display:
      | fprintf('Channel: %d',Channel)
      | port_label('input', 1, 'Warning signal ID')
      | port_label('input', 2, 'Maneuvre ID')
      | port_label('input', 3, 'Vehicle Type')
      | port_label('input', 4, 'Braking Capacity')
      | port_label('input', 5, 'Vehicle Size')
      | port_label('input', 6, 'spare')
  Constant : Constant  Value=80
  Constant1 : Constant  Value=50
  Constant2 : Constant  Value=80
  Control Lane2 : Subsystem
  Ground_{1..39} (39 alike) : Ground
  LANE 1 / LANE 3 : simulink/Signal Routing/Manual Switch  SystemSampleTime=-1  sw=0  action=0  varsize=off
  LANE 2 Open / Closed : simulink/Signal Routing/Manual Switch  SystemSampleTime=-1  sw=1  action=0  varsize=off
  Memory{1..6} (6 alike) : Memory
  Reinsert Vehicle Trigger  (time based) : DiscretePulseGenerator  PulseType=Time based  Period=3  PulseWidth=5
  "Reinsert Vehicle Trigger  (time based) " : DiscretePulseGenerator  PulseType=Time based  Period=3  PulseWidth=5
  Rounding Function{,1..5} (6 alike) : Rounding  Operator=round
  Status_Lane2 : Display
  Terminator_{1..36} (36 alike) : Terminator
  Throughput_Lane{1..6} (6 alike) : Display
  ini_cond4 : Constant  Value=3  SampleTime=0
  ini_cond6 : Constant  SampleTime=0
  ini_cond7 : Constant  Value=0  SampleTime=0
  Antenna_Tx_7 : Outport  Tag=AntennaTOutport
  Antenna_Tx_8 : Outport  Tag=AntennaTOutport  Port=2
  Antenna_Tx_9 : Outport  Tag=AntennaTOutport  Port=3
  tag scope: AdvisedVelocity_Lane2=global, Trigger_Lane1=global, Trigger_Lane3=global
  wires:
  [AdvisedVelocity_Lane1] -> Memory4/1
  [State_Lane2] -> Memory6/1
  [AdvisedVelocity_Lane2] -> Memory3/1
  [State_Lane2] -> Memory2/1
  [AdvisedVelocity_Lane3] -> Memory1/1
  [State_Lane2] -> Memory5/1
  [Velocity_Lane3] -> Rounding Function1/1
  [Velocity_Lane1] -> Rounding Function2/1
  ini_cond6/1 -> LANE 1 / LANE 3/1
  ini_cond4/1 -> LANE 1 / LANE 3/2
  LANE 1 / LANE 3/1 -> LANE 2 Open / Closed/1
  ini_cond7/1 -> LANE 2 Open / Closed/2
  LANE 2 Open / Closed/1 -> [IntendedState_Lane2]
  Reinsert Vehicle Trigger  (time based)/1 -> [Trigger_Lane1]
  "Reinsert Vehicle Trigger  (time based) "/1 -> [Trigger_Lane3]
  [Throughput_Lane1] -> Rounding Function3/1
  [Throughput_Lane3] -> Rounding Function/1
  [State_Lane2] -> Status_Lane2/1
  [Velocity_Lane2] -> Rounding Function4/1
  [Throughput_Lane2] -> Rounding Function5/1
  Constant/1 -> [AdvisedVelocity_Lane1]
  Constant1/1 -> [AdvisedVelocity_Lane2]
  Constant2/1 -> [AdvisedVelocity_Lane3]
  [IntendedState_Lane2] -> Control Lane2/1(Intended_State_Lane2 [0/1/3])
  Control Lane2/1(State Lane2 [0/1/3]) -> [State_Lane2]
  Rounding Function/1 -> Throughput_Lane3/1
  Rounding Function1/1 -> Throughput_Lane4/1
  Rounding Function2/1 -> Throughput_Lane6/1
  Rounding Function3/1 -> Throughput_Lane1/1
  Rounding Function4/1 -> Throughput_Lane5/1
  Rounding Function5/1 -> Throughput_Lane2/1
  Antenna_Rx_7_Demux/13(spare) -> Antenna_Rx_7_BusSelector/1
  Antenna_Rx_7/1 -> Antenna_Rx_7_Demux/1(AntennaR)
  Antenna_Tx_7_Mux/2(Out2) -> [Antenna_Tx_7]
  [Antenna_Tx_7] -> Antenna_Tx_7_BusAssignment/1
  Antenna_Tx_7_BusAssignment/1 -> Antenna_Tx_7_Mux/6(spare)
  Antenna_Tx_7_Mux/1(Out1) -> Antenna_Tx_7/1
  Antenna_Rx_8_Demux/13(spare) -> Antenna_Rx_8_BusSelector/1
  Antenna_Rx_8/1 -> Antenna_Rx_8_Demux/1(AntennaR)
  Antenna_Tx_8_Mux/2(Out2) -> [Antenna_Tx_8]
  [Antenna_Tx_8] -> Antenna_Tx_8_BusAssignment/1
  Antenna_Tx_8_BusAssignment/1 -> Antenna_Tx_8_Mux/6(spare)
  Antenna_Tx_8_Mux/1(Out1) -> Antenna_Tx_8/1
  Antenna_Rx_9_Demux/13(spare) -> Antenna_Rx_9_BusSelector/1
  Antenna_Rx_9/1 -> Antenna_Rx_9_Demux/1(AntennaR)
  Antenna_Tx_9_Mux/2(Out2) -> [Antenna_Tx_9]
  [Antenna_Tx_9] -> Antenna_Tx_9_BusAssignment/1
  Antenna_Tx_9_BusAssignment/1 -> Antenna_Tx_9_Mux/6(spare)
  Antenna_Tx_9_Mux/1(Out1) -> Antenna_Tx_9/1
  Memory4/1 -> Antenna_Tx_7_BusAssignment/4
  Memory6/1 -> Antenna_Tx_7_BusAssignment/5
  Memory2/1 -> Antenna_Tx_8_BusAssignment/5
  Memory3/1 -> Antenna_Tx_8_BusAssignment/4
  Memory1/1 -> Antenna_Tx_9_BusAssignment/4
  Memory5/1 -> Antenna_Tx_9_BusAssignment/5
  Antenna_Rx_7_BusSelector/1 -> [Velocity_Lane1]  "<Velocity_L1>"
  Antenna_Rx_7_BusSelector/2 -> [Throughput_Lane1]  "<Throughput_L1>"
  Antenna_Rx_8_BusSelector/1 -> [Velocity_Lane2]  "<Velocity_L2>"
  Antenna_Rx_8_BusSelector/2 -> [Throughput_Lane2]  "<Throughput_L2>"
  Antenna_Rx_9_BusSelector/1 -> [Velocity_Lane3]  "<Velocity_L3>"
  Antenna_Rx_9_BusSelector/2 -> [Throughput_Lane3]  "<Throughput_L3>"
  Antenna_Rx_7_Demux/1(Vehicle_ID) -> Terminator_1/1
  Antenna_Rx_7_Demux/2(GPS_pos_lat_[deg]) -> Terminator_2/1
  Antenna_Rx_7_Demux/3(GPS_pos_long_[deg]) -> Terminator_3/1
  Antenna_Rx_7_Demux/4(GPS_pos_alt_[m]) -> Terminator_4/1
  Antenna_Rx_7_Demux/5(Velocity_[m/s]) -> Terminator_5/1
  Antenna_Rx_7_Demux/6(Acceleration_[m/s2]) -> Terminator_6/1
  Antenna_Rx_7_Demux/7(Heading [deg]) -> Terminator_7/1
  Antenna_Rx_7_Demux/8(warning_signal_id) -> Terminator_8/1
  Antenna_Rx_7_Demux/9(maneuvre_id) -> Terminator_9/1
  Antenna_Rx_7_Demux/10(vehicle_type) -> Terminator_10/1
  Antenna_Rx_7_Demux/11(brakingcapacity) -> Terminator_11/1
  Antenna_Rx_7_Demux/12(vehicle_size) -> Terminator_12/1
  Antenna_Rx_8_Demux/1(Vehicle_ID) -> Terminator_13/1
  Antenna_Rx_8_Demux/2(GPS_pos_lat_[deg]) -> Terminator_14/1
  Antenna_Rx_8_Demux/3(GPS_pos_long_[deg]) -> Terminator_15/1
  Antenna_Rx_8_Demux/4(GPS_pos_alt_[m]) -> Terminator_16/1
  Antenna_Rx_8_Demux/5(Velocity_[m/s]) -> Terminator_17/1
  Antenna_Rx_8_Demux/6(Acceleration_[m/s2]) -> Terminator_18/1
  Antenna_Rx_8_Demux/7(Heading [deg]) -> Terminator_19/1
  Antenna_Rx_8_Demux/8(warning_signal_id) -> Terminator_20/1
  Antenna_Rx_8_Demux/9(maneuvre_id) -> Terminator_21/1
  Antenna_Rx_8_Demux/10(vehicle_type) -> Terminator_22/1
  Antenna_Rx_8_Demux/11(brakingcapacity) -> Terminator_23/1
  Antenna_Rx_8_Demux/12(vehicle_size) -> Terminator_24/1
  Antenna_Rx_9_Demux/1(Vehicle_ID) -> Terminator_25/1
  Antenna_Rx_9_Demux/2(GPS_pos_lat_[deg]) -> Terminator_26/1
  Antenna_Rx_9_Demux/3(GPS_pos_long_[deg]) -> Terminator_27/1
  Antenna_Rx_9_Demux/4(GPS_pos_alt_[m]) -> Terminator_28/1
  Antenna_Rx_9_Demux/5(Velocity_[m/s]) -> Terminator_29/1
  Antenna_Rx_9_Demux/6(Acceleration_[m/s2]) -> Terminator_30/1
  Antenna_Rx_9_Demux/7(Heading [deg]) -> Terminator_31/1
  Antenna_Rx_9_Demux/8(warning_signal_id) -> Terminator_32/1
  Antenna_Rx_9_Demux/9(maneuvre_id) -> Terminator_33/1
  Antenna_Rx_9_Demux/10(vehicle_type) -> Terminator_34/1
  Antenna_Rx_9_Demux/11(brakingcapacity) -> Terminator_35/1
  Antenna_Rx_9_Demux/12(vehicle_size) -> Terminator_36/1
  Ground_1/1 -> Antenna_Tx_7_BusAssignment/2
  Ground_2/1 -> Antenna_Tx_7_BusAssignment/3
  Ground_3/1 -> Antenna_Tx_7_BusAssignment/6
  Ground_4/1 -> Antenna_Tx_7_BusAssignment/7
  Ground_5/1 -> Antenna_Tx_7_BusAssignment/8
  Ground_6/1 -> Antenna_Tx_7_BusAssignment/9
  Ground_7/1 -> Antenna_Tx_7_BusAssignment/10
  Ground_8/1 -> Antenna_Tx_7_BusAssignment/11
  Ground_9/1 -> Antenna_Tx_7_Mux/1(warning_signal_id)
  Ground_10/1 -> Antenna_Tx_7_Mux/2(maneuvre_id)
  Ground_11/1 -> Antenna_Tx_7_Mux/3(vehicle_type)
  Ground_12/1 -> Antenna_Tx_7_Mux/4(brakingcapacity)
  Ground_13/1 -> Antenna_Tx_7_Mux/5(vehicle_size)
  Ground_14/1 -> Antenna_Tx_8_BusAssignment/2
  Ground_15/1 -> Antenna_Tx_8_BusAssignment/3
  Ground_16/1 -> Antenna_Tx_8_BusAssignment/6
  Ground_17/1 -> Antenna_Tx_8_BusAssignment/7
  Ground_18/1 -> Antenna_Tx_8_BusAssignment/8
  Ground_19/1 -> Antenna_Tx_8_BusAssignment/9
  Ground_20/1 -> Antenna_Tx_8_BusAssignment/10
  Ground_21/1 -> Antenna_Tx_8_BusAssignment/11
  Ground_22/1 -> Antenna_Tx_8_Mux/1(warning_signal_id)
  Ground_23/1 -> Antenna_Tx_8_Mux/2(maneuvre_id)
  Ground_24/1 -> Antenna_Tx_8_Mux/3(vehicle_type)
  Ground_25/1 -> Antenna_Tx_8_Mux/4(brakingcapacity)
  Ground_26/1 -> Antenna_Tx_8_Mux/5(vehicle_size)
  Ground_27/1 -> Antenna_Tx_9_BusAssignment/2
  Ground_28/1 -> Antenna_Tx_9_BusAssignment/3
  Ground_29/1 -> Antenna_Tx_9_BusAssignment/6
  Ground_30/1 -> Antenna_Tx_9_BusAssignment/7
  Ground_31/1 -> Antenna_Tx_9_BusAssignment/8
  Ground_32/1 -> Antenna_Tx_9_BusAssignment/9
  Ground_33/1 -> Antenna_Tx_9_BusAssignment/10
  Ground_34/1 -> Antenna_Tx_9_BusAssignment/11
  Ground_35/1 -> Antenna_Tx_9_Mux/1(warning_signal_id)
  Ground_36/1 -> Antenna_Tx_9_Mux/2(maneuvre_id)
  Ground_37/1 -> Antenna_Tx_9_Mux/3(vehicle_type)
  Ground_38/1 -> Antenna_Tx_9_Mux/4(brakingcapacity)
  Ground_39/1 -> Antenna_Tx_9_Mux/5(vehicle_size)

== system Demo_TrafficFlow_cs/GetSensors ==
  dummy : Inport
  GetSensors : M-S-Function  FunctionName=GetSensorsBlock  Parameters=TimeStep NrOfSensors
  tag scope: SimCoreSensors=global
  wires:
  GetSensors/1 -> [SimCoreSensors]
  dummy/1 -> GetSensors/1
